simulink model "ol_simulation"  (R2023b)
settings: Solver=FixedStepAuto  StartTime=0.0  StopTime=45  FixedStep=0.05  RelTol=1e-3
model workspace (MAT data in the model file):
  Logfile_Data_Object : robotics.ros.BagSelection (value not decoded)
  Logfile_Path = '/Users/sprinkle/work/teaching/cs3891-2023F/src/docker/mytest.bag'
  Logfile_Playback_Duration = 200
  Logfile_Playback_Offset = 100
libraries: simulink x1
settings Model: Creator=sprinkle  LastModifiedBy=marystirlingbrown
settings SolverCC: AlgebraicLoopSolver=TrustRegion
settings OptimizationCC: EnhancedBackFolding=on  PassReuseOutputArgsAs=Individual arguments  ZeroExternalMemoryAtStartup=off  ZeroInternalMemoryAtStartup=off  MaxStackSize=64  OptimizeBlockOrder=speed  DifferentSizesBufferReuse=on  OptimizationCustomize=off  EfficientTunableParamExpr=on
settings DebuggingCC: ParameterTunabilityLossMsg=error  UnconnectedInputMsg=warning  UnconnectedOutputMsg=warning  UnconnectedLineMsg=warning
settings HardwareCC: ProdBitPerPointer=32  ProdBitPerSizeT=32  ProdBitPerPtrDiffT=32  ProdLargestAtomicFloat=None  ProdIntDivRoundTo=Undefined  ProdEndianess=Unspecified  ProdWordSize=32  ProdHWDeviceType=Generic->Unspecified (assume 32-bit Generic)
settings ModelReferenceCC: EnableRefExpFcnMdlSchedulingChecks=on
settings SFSimCC: MATLABDynamicMemAlloc=off
settings RTWCC: Description=Embedded Coder  SystemTargetFile=ert.tlc  HardwareBoard=Robot Operating System (ROS)  TLCOptions="-aInlineSetEventsForThisBaseRateFcn=TLC_FALSE -aSuppressMultiTaskScheduler=TLC_FALSE  -aRateBasedStepFcn=0"  TemplateMakefile=ert_default_tmf  Toolchain=Catkin  TargetLang=C++
settings CodeAppCC: ForceParamTrailComments=on  IgnoreCustomStorageClasses=off  OperatorAnnotations=on  SimulinkDataObjDesc=on  SFDataObjDesc=on  InsertBlockDesc=on
settings ERTTargetCC: SupportContinuousTime=on

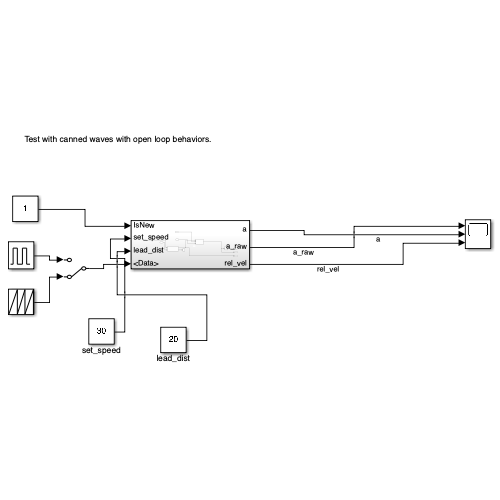
[thumbnail: Simulink's stored preview of the top-level diagram]
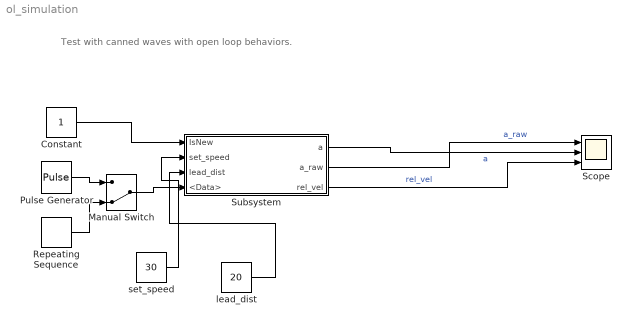
== system ol_simulation ==
  note: Test with canned waves with open loop behaviors.
  Constant : Constant
  Manual Switch : ManualSwitch  CurrentSetting=0
  Pulse Generator : DiscretePulseGenerator  PulseType=Time based  Amplitude=2  Period=10  PulseWidth=30
  Repeating Sequence : simulink/Sources/Repeating Sequence  rep_seq_t=[0 20]  rep_seq_y=[-2 2]
  Scope : Scope  NumInputPorts=3
  Subsystem : Subsystem
  lead_dist : Constant  Value=20
  set_speed : Constant  Value=30
  wires:
  Subsystem/1(a) -> Scope/2  "a"
  Subsystem/2(a_raw) -> Scope/1  "a_raw"
  Subsystem/3(rel_vel) -> Scope/3  "rel_vel"
  Constant/1 -> Subsystem/1(IsNew)
  Manual Switch/1 -> Subsystem/4(<Data>)
  Pulse Generator/1 -> Manual Switch/1
  Repeating Sequence/1 -> Manual Switch/2
  set_speed/1 -> Subsystem/2(set_speed)
  lead_dist/1 -> Subsystem/3(lead_dist)

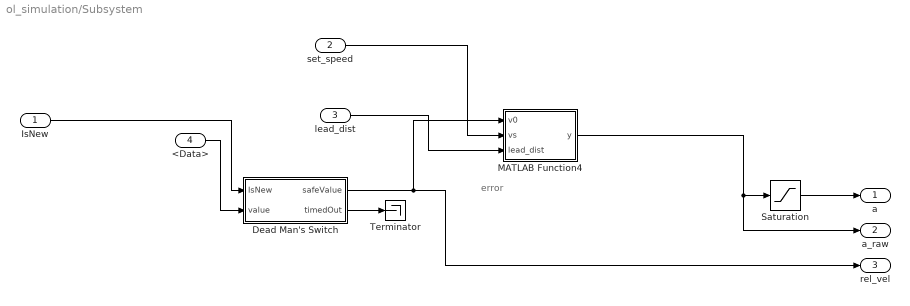
== system ol_simulation/Subsystem ==
  note: error
  IsNew : Inport
  set_speed : Inport  Port=2
  lead_dist : Inport  Port=3
  <Data> : Inport  Port=4
  Dead Man's Switch : Subsystem  TreatAsAtomicUnit=on  mask{stepSize=0.05, timeout=0.4}
      mask prompts: stepSize "Simulation step size"; timeout "Timeout (in seconds)"
      mask help: Interprets whether a new message has been recently received, and if so permits the data in 'value' to be passed through. Otherwise, 0 is outputted from 'safeValue'
      mask Help: Interprets whether a new message has been recently received, and if so permits the data in 'value' to be passed through. Otherwise, 0 is outputted from 'safeValue'
  MATLAB Function4 : MATLAB Function  TreatAsAtomicUnit=on
  Saturation : Saturate  UpperLimit=1.5  LowerLimit=-3
  Terminator : Terminator
  a : Outport
  a_raw : Outport  Port=2
  rel_vel : Outport  Port=3
  wires:
  IsNew/1 -> Dead Man's Switch/1(IsNew)
  Dead Man's Switch/2(timedOut) -> Terminator/1
  <Data>/1 -> Dead Man's Switch/2(value)
  Saturation/1 -> a/1
  Dead Man's Switch/1(safeValue) -> MATLAB Function4/1(v0), rel_vel/1
  MATLAB Function4/1(y) -> a_raw/1, Saturation/1
  lead_dist/1 -> MATLAB Function4/3(lead_dist)
  set_speed/1 -> MATLAB Function4/2(vs)

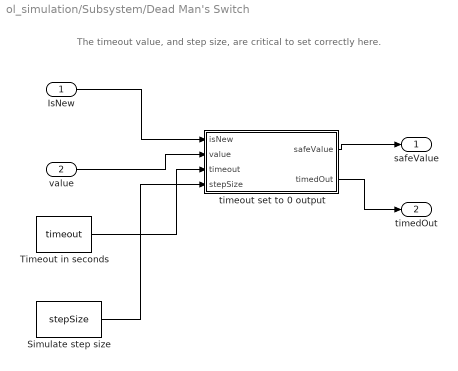
== system ol_simulation/Subsystem/Dead Man's Switch ==
  note: The timeout value, and step size, are critical to set correctly here.
  IsNew : Inport
  value : Inport  Port=2
  Simulate step size : Constant  Value=stepSize
  Timeout in seconds : Constant  Value=timeout
  timeout set to 0 output : MATLAB Function  TreatAsAtomicUnit=on
  safeValue : Outport  InitialOutput=[0]
  timedOut : Outport  Port=2  InitialOutput=[0]
  wires:
  IsNew/1 -> timeout set to 0 output/1(isNew)
  timeout set to 0 output/1(safeValue) -> safeValue/1
  value/1 -> timeout set to 0 output/2(value)
  Timeout in seconds/1 -> timeout set to 0 output/3(timeout)
  timeout set to 0 output/2(timedOut) -> timedOut/1
  Simulate step size/1 -> timeout set to 0 output/4(stepSize)

== matlab function ol_simulation/Subsystem/Dead Man's Switch/timeout set to 0 output ==
  settings: updateMethod=DISCRETE
  data: isNew input; safeValue output; value input; timeout input; timedOut output; stepSize input
function [safeValue,timedOut] = fcn(isNew,value,timeout,stepSize)
%#codegen
persistent sinceLastMsg
timedOut=true;
if isempty(sinceLastMsg)
    sinceLastMsg=(timeout/stepSize)+1;
end

safeValue=0;
% if no new message
% after timeout, we output 0
if( isNew == true )
    sinceLastMsg = 0;
else
    sinceLastMsg = sinceLastMsg+1;
end

% Note: we require step size as an input here, and depend on our
% system being executed at a regular rate, or bad things will happen
if( sinceLastMsg < timeout/stepSize )
    timedOut = false;
end
    
if( timedOut == false )
    safeValue = value;
end

== matlab function ol_simulation/Subsystem/MATLAB Function4 ==
  data: v0 input; y output; vs input; lead_dist input
function y = fcn(v0, vs, lead_dist)

    y = 0; % Default value if vs is equal to v0

    if vs ~= v0
        y = accel(v0, vs, lead_dist);
    end
end

function acceleration = accel(V_0, V_S, lead_dist)
   
    % Calculate time gap
    T_g = lead_dist / V_0;

    if T_g < 2
        acceleration = 0;
        return;
    end

    if V_S > V_0
        % Check safety and apply acceleration
        if ~safe(T_g)
            acceleration = 0;
        end
        % Calculate acceleration based on an arbitrary constant
        acceleration = (V_S - V_0) / 10;
        
        
    elseif V_S < V_0
        
        % Calculate deceleration based on an arbitrary constant
        acceleration = (V_S - V_0) / 10;
    else
        % If V_S is equal to V_0, no acceleration or deceleration
        acceleration = 0;
    end

end

function result = safe(Tau)
    % Check if the time gap is less than 2 seconds
    if Tau < 2
        result = false;
    else
        result = true;
    end
end
